simulink model "wind1000_BUS_Original"  (R2024a)
settings: Solver=ode45  StartTime=parSimulacio.ti  StopTime=40  MaxStep=parSimulacio.pas  RelTol=1e-6  AbsTol=1e-6  EnableMultiTasking=on
model workspace (MAT data in the model file):
  tt_vw : Simulink.Parameter (value not decoded)
values from neighbouring files (symbols this model uses):
  L = L'    [parameters_windFarm2.m]
  nu = size(S, 1)    [parameters_windFarm2.m]
undefined symbols (not defined in the file or in its folder): parControl, parConvertidor, parGenerador, parGrid, parMecanica, parSimulacio, S, w0, windspeed
(part 6 of 218)

== system wind1000_BUS_Original/Subsystem_200/Subsystem/String16/WT1 ==
  V_in : Inport
  I_in : Inport  Port=2
  Add1 : Sum  Inputs=+-
  C_cable : Subsystem
  C_filter : Subsystem
  Cable line : Subsystem
  Gain : Gain  Gain=1/parGrid.WTstring.Rparallel_cable
  Transformer-complex : Subsystem
  WFG : Subsystem
  V_out : Outport
  I_out : Outport  Port=2
  wires:
  I_in/1 -> C_cable/2(I_in)
  Gain/1 -> Add1/2
  Add1/1 -> C_cable/1(It2)
  Transformer-complex/2(It2) -> Add1/1
  V_in/1 -> Cable line/1(V)
  Transformer-complex/1(It1) -> C_filter/2(It1)
  Cable line/1(I_cable) -> C_cable/3(I_cable), I_out/1
  C_filter/1(Vc_filter_qd) -> WFG/1(vcqd), Transformer-complex/1(Vc_filter_qd)
  WFG/1(ilqd) -> C_filter/1(IWF)
  C_cable/1(Vc_cable) -> Cable line/2(Vc_cable), V_out/1, Transformer-complex/2(Vc_cable_qd), Gain/1

== system wind1000_BUS_Original/Subsystem_200/Subsystem/String16/WT10 ==
  V_in : Inport
  I_in : Inport  Port=2
  Add1 : Sum  Inputs=+-
  C_cable : Subsystem
  C_filter : Subsystem
  Cable line : Subsystem
  Gain : Gain  Gain=1/parGrid.WTstring.Rparallel_cable
  Transformer-complex : Subsystem
  WFG : Subsystem
  V_out : Outport
  I_out : Outport  Port=2
  wires:
  C_cable/1(Vc_cable) -> Gain/1, Transformer-complex/2(Vc_cable_qd), V_out/1, Cable line/2(Vc_cable)
  WFG/1(ilqd) -> C_filter/1(IWF)
  C_filter/1(Vc_filter_qd) -> Transformer-complex/1(Vc_filter_qd), WFG/1(vcqd)
  Cable line/1(I_cable) -> I_out/1, C_cable/3(I_cable)
  Transformer-complex/1(It1) -> C_filter/2(It1)
  V_in/1 -> Cable line/1(V)
  Transformer-complex/2(It2) -> Add1/1
  Add1/1 -> C_cable/1(It2)
  Gain/1 -> Add1/2
  I_in/1 -> C_cable/2(I_in)

== system wind1000_BUS_Original/Subsystem_200/Subsystem/String16/WT2 ==
  V_in : Inport
  I_in : Inport  Port=2
  Add1 : Sum  Inputs=+-
  C_cable : Subsystem
  C_filter : Subsystem
  Cable line : Subsystem
  Gain : Gain  Gain=1/parGrid.WTstring.Rparallel_cable
  Transformer-complex : Subsystem
  WFG : Subsystem
  V_out : Outport
  I_out : Outport  Port=2
  wires:
  C_cable/1(Vc_cable) -> Gain/1, Transformer-complex/2(Vc_cable_qd), V_out/1, Cable line/2(Vc_cable)
  WFG/1(ilqd) -> C_filter/1(IWF)
  C_filter/1(Vc_filter_qd) -> Transformer-complex/1(Vc_filter_qd), WFG/1(vcqd)
  Cable line/1(I_cable) -> I_out/1, C_cable/3(I_cable)
  Transformer-complex/1(It1) -> C_filter/2(It1)
  V_in/1 -> Cable line/1(V)
  Transformer-complex/2(It2) -> Add1/1
  Add1/1 -> C_cable/1(It2)
  Gain/1 -> Add1/2
  I_in/1 -> C_cable/2(I_in)

== system wind1000_BUS_Original/Subsystem_200/Subsystem/String16/WT3 ==
  V_in : Inport
  I_in : Inport  Port=2
  Add1 : Sum  Inputs=+-
  C_cable : Subsystem
  C_filter : Subsystem
  Cable line : Subsystem
  Gain : Gain  Gain=1/parGrid.WTstring.Rparallel_cable
  Transformer-complex : Subsystem
  WFG : Subsystem
  V_out : Outport
  I_out : Outport  Port=2
  wires:
  I_in/1 -> C_cable/2(I_in)
  Gain/1 -> Add1/2
  Add1/1 -> C_cable/1(It2)
  Transformer-complex/2(It2) -> Add1/1
  V_in/1 -> Cable line/1(V)
  Transformer-complex/1(It1) -> C_filter/2(It1)
  Cable line/1(I_cable) -> C_cable/3(I_cable), I_out/1
  C_filter/1(Vc_filter_qd) -> Transformer-complex/1(Vc_filter_qd), WFG/1(vcqd)
  WFG/1(ilqd) -> C_filter/1(IWF)
  C_cable/1(Vc_cable) -> Cable line/2(Vc_cable), V_out/1, Transformer-complex/2(Vc_cable_qd), Gain/1

== system wind1000_BUS_Original/Subsystem_200/Subsystem/String16/WT4 ==
  V_in : Inport
  I_in : Inport  Port=2
  Add1 : Sum  Inputs=+-
  C_cable : Subsystem
  C_filter : Subsystem
  Cable line : Subsystem
  Gain : Gain  Gain=1/parGrid.WTstring.Rparallel_cable
  Transformer-complex : Subsystem
  WFG : Subsystem
  V_out : Outport
  I_out : Outport  Port=2
  wires:
  C_cable/1(Vc_cable) -> Gain/1, Transformer-complex/2(Vc_cable_qd), V_out/1, Cable line/2(Vc_cable)
  WFG/1(ilqd) -> C_filter/1(IWF)
  C_filter/1(Vc_filter_qd) -> Transformer-complex/1(Vc_filter_qd), WFG/1(vcqd)
  Cable line/1(I_cable) -> I_out/1, C_cable/3(I_cable)
  Transformer-complex/1(It1) -> C_filter/2(It1)
  V_in/1 -> Cable line/1(V)
  Transformer-complex/2(It2) -> Add1/1
  Add1/1 -> C_cable/1(It2)
  Gain/1 -> Add1/2
  I_in/1 -> C_cable/2(I_in)

== system wind1000_BUS_Original/Subsystem_200/Subsystem/String16/WT5 ==
  V_in : Inport
  I_in : Inport  Port=2
  Add1 : Sum  Inputs=+-
  C_cable : Subsystem
  C_filter : Subsystem
  Cable line : Subsystem
  Gain : Gain  Gain=1/parGrid.WTstring.Rparallel_cable
  Transformer-complex : Subsystem
  WFG : Subsystem
  V_out : Outport
  I_out : Outport  Port=2
  wires:
  I_in/1 -> C_cable/2(I_in)
  Gain/1 -> Add1/2
  Add1/1 -> C_cable/1(It2)
  Transformer-complex/2(It2) -> Add1/1
  V_in/1 -> Cable line/1(V)
  Transformer-complex/1(It1) -> C_filter/2(It1)
  Cable line/1(I_cable) -> C_cable/3(I_cable), I_out/1
  C_filter/1(Vc_filter_qd) -> Transformer-complex/1(Vc_filter_qd), WFG/1(vcqd)
  WFG/1(ilqd) -> C_filter/1(IWF)
  C_cable/1(Vc_cable) -> Cable line/2(Vc_cable), V_out/1, Transformer-complex/2(Vc_cable_qd), Gain/1

== system wind1000_BUS_Original/Subsystem_200/Subsystem/String16/WT6 ==
  V_in : Inport
  I_in : Inport  Port=2
  Add1 : Sum  Inputs=+-
  C_cable : Subsystem
  C_filter : Subsystem
  Cable line : Subsystem
  Gain : Gain  Gain=1/parGrid.WTstring.Rparallel_cable
  Transformer-complex : Subsystem
  WFG : Subsystem
  V_out : Outport
  I_out : Outport  Port=2
  wires:
  C_cable/1(Vc_cable) -> Gain/1, Transformer-complex/2(Vc_cable_qd), V_out/1, Cable line/2(Vc_cable)
  WFG/1(ilqd) -> C_filter/1(IWF)
  C_filter/1(Vc_filter_qd) -> Transformer-complex/1(Vc_filter_qd), WFG/1(vcqd)
  Cable line/1(I_cable) -> I_out/1, C_cable/3(I_cable)
  Transformer-complex/1(It1) -> C_filter/2(It1)
  V_in/1 -> Cable line/1(V)
  Transformer-complex/2(It2) -> Add1/1
  Add1/1 -> C_cable/1(It2)
  Gain/1 -> Add1/2
  I_in/1 -> C_cable/2(I_in)

== system wind1000_BUS_Original/Subsystem_200/Subsystem/String16/WT7 ==
  V_in : Inport
  I_in : Inport  Port=2
  Add1 : Sum  Inputs=+-
  C_cable : Subsystem
  C_filter : Subsystem
  Cable line : Subsystem
  Gain : Gain  Gain=1/parGrid.WTstring.Rparallel_cable
  Transformer-complex : Subsystem
  WFG : Subsystem
  V_out : Outport
  I_out : Outport  Port=2
  wires:
  I_in/1 -> C_cable/2(I_in)
  Gain/1 -> Add1/2
  Add1/1 -> C_cable/1(It2)
  Transformer-complex/2(It2) -> Add1/1
  V_in/1 -> Cable line/1(V)
  Transformer-complex/1(It1) -> C_filter/2(It1)
  Cable line/1(I_cable) -> C_cable/3(I_cable), I_out/1
  C_filter/1(Vc_filter_qd) -> Transformer-complex/1(Vc_filter_qd), WFG/1(vcqd)
  WFG/1(ilqd) -> C_filter/1(IWF)
  C_cable/1(Vc_cable) -> Cable line/2(Vc_cable), V_out/1, Transformer-complex/2(Vc_cable_qd), Gain/1

== system wind1000_BUS_Original/Subsystem_200/Subsystem/String16/WT8 ==
  V_in : Inport
  I_in : Inport  Port=2
  Add1 : Sum  Inputs=+-
  C_cable : Subsystem
  C_filter : Subsystem
  Cable line : Subsystem
  Gain : Gain  Gain=1/parGrid.WTstring.Rparallel_cable
  Transformer-complex : Subsystem
  WFG : Subsystem
  V_out : Outport
  I_out : Outport  Port=2
  wires:
  C_cable/1(Vc_cable) -> Gain/1, Transformer-complex/2(Vc_cable_qd), V_out/1, Cable line/2(Vc_cable)
  WFG/1(ilqd) -> C_filter/1(IWF)
  C_filter/1(Vc_filter_qd) -> Transformer-complex/1(Vc_filter_qd), WFG/1(vcqd)
  Cable line/1(I_cable) -> I_out/1, C_cable/3(I_cable)
  Transformer-complex/1(It1) -> C_filter/2(It1)
  V_in/1 -> Cable line/1(V)
  Transformer-complex/2(It2) -> Add1/1
  Add1/1 -> C_cable/1(It2)
  Gain/1 -> Add1/2
  I_in/1 -> C_cable/2(I_in)

== system wind1000_BUS_Original/Subsystem_200/Subsystem/String16/WT9 ==
  V_in : Inport
  I_in : Inport  Port=2
  Add1 : Sum  Inputs=+-
  C_cable : Subsystem
  C_filter : Subsystem
  Cable line : Subsystem
  Gain : Gain  Gain=1/parGrid.WTstring.Rparallel_cable
  Transformer-complex : Subsystem
  WFG : Subsystem
  V_out : Outport
  I_out : Outport  Port=2
  wires:
  I_in/1 -> C_cable/2(I_in)
  Gain/1 -> Add1/2
  Add1/1 -> C_cable/1(It2)
  Transformer-complex/2(It2) -> Add1/1
  V_in/1 -> Cable line/1(V)
  Transformer-complex/1(It1) -> C_filter/2(It1)
  Cable line/1(I_cable) -> C_cable/3(I_cable), I_out/1
  C_filter/1(Vc_filter_qd) -> Transformer-complex/1(Vc_filter_qd), WFG/1(vcqd)
  WFG/1(ilqd) -> C_filter/1(IWF)
  C_cable/1(Vc_cable) -> Cable line/2(Vc_cable), V_out/1, Transformer-complex/2(Vc_cable_qd), Gain/1

== system wind1000_BUS_Original/Subsystem_200/Subsystem/String17/WT1 ==
  V_in : Inport
  I_in : Inport  Port=2
  Add1 : Sum  Inputs=+-
  C_cable : Subsystem
  C_filter : Subsystem
  Cable line : Subsystem
  Gain : Gain  Gain=1/parGrid.WTstring.Rparallel_cable
  Transformer-complex : Subsystem
  WFG : Subsystem
  V_out : Outport
  I_out : Outport  Port=2
  wires:
  I_in/1 -> C_cable/2(I_in)
  Gain/1 -> Add1/2
  Add1/1 -> C_cable/1(It2)
  Transformer-complex/2(It2) -> Add1/1
  V_in/1 -> Cable line/1(V)
  Transformer-complex/1(It1) -> C_filter/2(It1)
  Cable line/1(I_cable) -> C_cable/3(I_cable), I_out/1
  C_filter/1(Vc_filter_qd) -> WFG/1(vcqd), Transformer-complex/1(Vc_filter_qd)
  WFG/1(ilqd) -> C_filter/1(IWF)
  C_cable/1(Vc_cable) -> Cable line/2(Vc_cable), V_out/1, Transformer-complex/2(Vc_cable_qd), Gain/1

== system wind1000_BUS_Original/Subsystem_200/Subsystem/String17/WT10 ==
  V_in : Inport
  I_in : Inport  Port=2
  Add1 : Sum  Inputs=+-
  C_cable : Subsystem
  C_filter : Subsystem
  Cable line : Subsystem
  Gain : Gain  Gain=1/parGrid.WTstring.Rparallel_cable
  Transformer-complex : Subsystem
  WFG : Subsystem
  V_out : Outport
  I_out : Outport  Port=2
  wires:
  C_cable/1(Vc_cable) -> Gain/1, Transformer-complex/2(Vc_cable_qd), V_out/1, Cable line/2(Vc_cable)
  WFG/1(ilqd) -> C_filter/1(IWF)
  C_filter/1(Vc_filter_qd) -> Transformer-complex/1(Vc_filter_qd), WFG/1(vcqd)
  Cable line/1(I_cable) -> I_out/1, C_cable/3(I_cable)
  Transformer-complex/1(It1) -> C_filter/2(It1)
  V_in/1 -> Cable line/1(V)
  Transformer-complex/2(It2) -> Add1/1
  Add1/1 -> C_cable/1(It2)
  Gain/1 -> Add1/2
  I_in/1 -> C_cable/2(I_in)

== system wind1000_BUS_Original/Subsystem_200/Subsystem/String17/WT2 ==
  V_in : Inport
  I_in : Inport  Port=2
  Add1 : Sum  Inputs=+-
  C_cable : Subsystem
  C_filter : Subsystem
  Cable line : Subsystem
  Gain : Gain  Gain=1/parGrid.WTstring.Rparallel_cable
  Transformer-complex : Subsystem
  WFG : Subsystem
  V_out : Outport
  I_out : Outport  Port=2
  wires:
  C_cable/1(Vc_cable) -> Gain/1, Transformer-complex/2(Vc_cable_qd), V_out/1, Cable line/2(Vc_cable)
  WFG/1(ilqd) -> C_filter/1(IWF)
  C_filter/1(Vc_filter_qd) -> Transformer-complex/1(Vc_filter_qd), WFG/1(vcqd)
  Cable line/1(I_cable) -> I_out/1, C_cable/3(I_cable)
  Transformer-complex/1(It1) -> C_filter/2(It1)
  V_in/1 -> Cable line/1(V)
  Transformer-complex/2(It2) -> Add1/1
  Add1/1 -> C_cable/1(It2)
  Gain/1 -> Add1/2
  I_in/1 -> C_cable/2(I_in)

== system wind1000_BUS_Original/Subsystem_200/Subsystem/String17/WT3 ==
  V_in : Inport
  I_in : Inport  Port=2
  Add1 : Sum  Inputs=+-
  C_cable : Subsystem
  C_filter : Subsystem
  Cable line : Subsystem
  Gain : Gain  Gain=1/parGrid.WTstring.Rparallel_cable
  Transformer-complex : Subsystem
  WFG : Subsystem
  V_out : Outport
  I_out : Outport  Port=2
  wires:
  I_in/1 -> C_cable/2(I_in)
  Gain/1 -> Add1/2
  Add1/1 -> C_cable/1(It2)
  Transformer-complex/2(It2) -> Add1/1
  V_in/1 -> Cable line/1(V)
  Transformer-complex/1(It1) -> C_filter/2(It1)
  Cable line/1(I_cable) -> C_cable/3(I_cable), I_out/1
  C_filter/1(Vc_filter_qd) -> Transformer-complex/1(Vc_filter_qd), WFG/1(vcqd)
  WFG/1(ilqd) -> C_filter/1(IWF)
  C_cable/1(Vc_cable) -> Cable line/2(Vc_cable), V_out/1, Transformer-complex/2(Vc_cable_qd), Gain/1

== system wind1000_BUS_Original/Subsystem_200/Subsystem/String17/WT4 ==
  V_in : Inport
  I_in : Inport  Port=2
  Add1 : Sum  Inputs=+-
  C_cable : Subsystem
  C_filter : Subsystem
  Cable line : Subsystem
  Gain : Gain  Gain=1/parGrid.WTstring.Rparallel_cable
  Transformer-complex : Subsystem
  WFG : Subsystem
  V_out : Outport
  I_out : Outport  Port=2
  wires:
  C_cable/1(Vc_cable) -> Gain/1, Transformer-complex/2(Vc_cable_qd), V_out/1, Cable line/2(Vc_cable)
  WFG/1(ilqd) -> C_filter/1(IWF)
  C_filter/1(Vc_filter_qd) -> Transformer-complex/1(Vc_filter_qd), WFG/1(vcqd)
  Cable line/1(I_cable) -> I_out/1, C_cable/3(I_cable)
  Transformer-complex/1(It1) -> C_filter/2(It1)
  V_in/1 -> Cable line/1(V)
  Transformer-complex/2(It2) -> Add1/1
  Add1/1 -> C_cable/1(It2)
  Gain/1 -> Add1/2
  I_in/1 -> C_cable/2(I_in)

== system wind1000_BUS_Original/Subsystem_200/Subsystem/String17/WT5 ==
  V_in : Inport
  I_in : Inport  Port=2
  Add1 : Sum  Inputs=+-
  C_cable : Subsystem
  C_filter : Subsystem
  Cable line : Subsystem
  Gain : Gain  Gain=1/parGrid.WTstring.Rparallel_cable
  Transformer-complex : Subsystem
  WFG : Subsystem
  V_out : Outport
  I_out : Outport  Port=2
  wires:
  I_in/1 -> C_cable/2(I_in)
  Gain/1 -> Add1/2
  Add1/1 -> C_cable/1(It2)
  Transformer-complex/2(It2) -> Add1/1
  V_in/1 -> Cable line/1(V)
  Transformer-complex/1(It1) -> C_filter/2(It1)
  Cable line/1(I_cable) -> C_cable/3(I_cable), I_out/1
  C_filter/1(Vc_filter_qd) -> Transformer-complex/1(Vc_filter_qd), WFG/1(vcqd)
  WFG/1(ilqd) -> C_filter/1(IWF)
  C_cable/1(Vc_cable) -> Cable line/2(Vc_cable), V_out/1, Transformer-complex/2(Vc_cable_qd), Gain/1

== system wind1000_BUS_Original/Subsystem_200/Subsystem/String17/WT6 ==
  V_in : Inport
  I_in : Inport  Port=2
  Add1 : Sum  Inputs=+-
  C_cable : Subsystem
  C_filter : Subsystem
  Cable line : Subsystem
  Gain : Gain  Gain=1/parGrid.WTstring.Rparallel_cable
  Transformer-complex : Subsystem
  WFG : Subsystem
  V_out : Outport
  I_out : Outport  Port=2
  wires:
  C_cable/1(Vc_cable) -> Gain/1, Transformer-complex/2(Vc_cable_qd), V_out/1, Cable line/2(Vc_cable)
  WFG/1(ilqd) -> C_filter/1(IWF)
  C_filter/1(Vc_filter_qd) -> Transformer-complex/1(Vc_filter_qd), WFG/1(vcqd)
  Cable line/1(I_cable) -> I_out/1, C_cable/3(I_cable)
  Transformer-complex/1(It1) -> C_filter/2(It1)
  V_in/1 -> Cable line/1(V)
  Transformer-complex/2(It2) -> Add1/1
  Add1/1 -> C_cable/1(It2)
  Gain/1 -> Add1/2
  I_in/1 -> C_cable/2(I_in)

== system wind1000_BUS_Original/Subsystem_200/Subsystem/String17/WT7 ==
  V_in : Inport
  I_in : Inport  Port=2
  Add1 : Sum  Inputs=+-
  C_cable : Subsystem
  C_filter : Subsystem
  Cable line : Subsystem
  Gain : Gain  Gain=1/parGrid.WTstring.Rparallel_cable
  Transformer-complex : Subsystem
  WFG : Subsystem
  V_out : Outport
  I_out : Outport  Port=2
  wires:
  I_in/1 -> C_cable/2(I_in)
  Gain/1 -> Add1/2
  Add1/1 -> C_cable/1(It2)
  Transformer-complex/2(It2) -> Add1/1
  V_in/1 -> Cable line/1(V)
  Transformer-complex/1(It1) -> C_filter/2(It1)
  Cable line/1(I_cable) -> C_cable/3(I_cable), I_out/1
  C_filter/1(Vc_filter_qd) -> Transformer-complex/1(Vc_filter_qd), WFG/1(vcqd)
  WFG/1(ilqd) -> C_filter/1(IWF)
  C_cable/1(Vc_cable) -> Cable line/2(Vc_cable), V_out/1, Transformer-complex/2(Vc_cable_qd), Gain/1

== system wind1000_BUS_Original/Subsystem_200/Subsystem/String17/WT8 ==
  V_in : Inport
  I_in : Inport  Port=2
  Add1 : Sum  Inputs=+-
  C_cable : Subsystem
  C_filter : Subsystem
  Cable line : Subsystem
  Gain : Gain  Gain=1/parGrid.WTstring.Rparallel_cable
  Transformer-complex : Subsystem
  WFG : Subsystem
  V_out : Outport
  I_out : Outport  Port=2
  wires:
  C_cable/1(Vc_cable) -> Gain/1, Transformer-complex/2(Vc_cable_qd), V_out/1, Cable line/2(Vc_cable)
  WFG/1(ilqd) -> C_filter/1(IWF)
  C_filter/1(Vc_filter_qd) -> Transformer-complex/1(Vc_filter_qd), WFG/1(vcqd)
  Cable line/1(I_cable) -> I_out/1, C_cable/3(I_cable)
  Transformer-complex/1(It1) -> C_filter/2(It1)
  V_in/1 -> Cable line/1(V)
  Transformer-complex/2(It2) -> Add1/1
  Add1/1 -> C_cable/1(It2)
  Gain/1 -> Add1/2
  I_in/1 -> C_cable/2(I_in)

== system wind1000_BUS_Original/Subsystem_200/Subsystem/String17/WT9 ==
  V_in : Inport
  I_in : Inport  Port=2
  Add1 : Sum  Inputs=+-
  C_cable : Subsystem
  C_filter : Subsystem
  Cable line : Subsystem
  Gain : Gain  Gain=1/parGrid.WTstring.Rparallel_cable
  Transformer-complex : Subsystem
  WFG : Subsystem
  V_out : Outport
  I_out : Outport  Port=2
  wires:
  I_in/1 -> C_cable/2(I_in)
  Gain/1 -> Add1/2
  Add1/1 -> C_cable/1(It2)
  Transformer-complex/2(It2) -> Add1/1
  V_in/1 -> Cable line/1(V)
  Transformer-complex/1(It1) -> C_filter/2(It1)
  Cable line/1(I_cable) -> C_cable/3(I_cable), I_out/1
  C_filter/1(Vc_filter_qd) -> Transformer-complex/1(Vc_filter_qd), WFG/1(vcqd)
  WFG/1(ilqd) -> C_filter/1(IWF)
  C_cable/1(Vc_cable) -> Cable line/2(Vc_cable), V_out/1, Transformer-complex/2(Vc_cable_qd), Gain/1

== system wind1000_BUS_Original/Subsystem_200/Subsystem/String18/WT1 ==
  V_in : Inport
  I_in : Inport  Port=2
  Add1 : Sum  Inputs=+-
  C_cable : Subsystem
  C_filter : Subsystem
  Cable line : Subsystem
  Gain : Gain  Gain=1/parGrid.WTstring.Rparallel_cable
  Transformer-complex : Subsystem
  WFG : Subsystem
  V_out : Outport
  I_out : Outport  Port=2
  wires:
  I_in/1 -> C_cable/2(I_in)
  Gain/1 -> Add1/2
  Add1/1 -> C_cable/1(It2)
  Transformer-complex/2(It2) -> Add1/1
  V_in/1 -> Cable line/1(V)
  Transformer-complex/1(It1) -> C_filter/2(It1)
  Cable line/1(I_cable) -> C_cable/3(I_cable), I_out/1
  C_filter/1(Vc_filter_qd) -> WFG/1(vcqd), Transformer-complex/1(Vc_filter_qd)
  WFG/1(ilqd) -> C_filter/1(IWF)
  C_cable/1(Vc_cable) -> Cable line/2(Vc_cable), V_out/1, Transformer-complex/2(Vc_cable_qd), Gain/1

== system wind1000_BUS_Original/Subsystem_200/Subsystem/String18/WT10 ==
  V_in : Inport
  I_in : Inport  Port=2
  Add1 : Sum  Inputs=+-
  C_cable : Subsystem
  C_filter : Subsystem
  Cable line : Subsystem
  Gain : Gain  Gain=1/parGrid.WTstring.Rparallel_cable
  Transformer-complex : Subsystem
  WFG : Subsystem
  V_out : Outport
  I_out : Outport  Port=2
  wires:
  C_cable/1(Vc_cable) -> Gain/1, Transformer-complex/2(Vc_cable_qd), V_out/1, Cable line/2(Vc_cable)
  WFG/1(ilqd) -> C_filter/1(IWF)
  C_filter/1(Vc_filter_qd) -> Transformer-complex/1(Vc_filter_qd), WFG/1(vcqd)
  Cable line/1(I_cable) -> I_out/1, C_cable/3(I_cable)
  Transformer-complex/1(It1) -> C_filter/2(It1)
  V_in/1 -> Cable line/1(V)
  Transformer-complex/2(It2) -> Add1/1
  Add1/1 -> C_cable/1(It2)
  Gain/1 -> Add1/2
  I_in/1 -> C_cable/2(I_in)

== system wind1000_BUS_Original/Subsystem_200/Subsystem/String18/WT2 ==
  V_in : Inport
  I_in : Inport  Port=2
  Add1 : Sum  Inputs=+-
  C_cable : Subsystem
  C_filter : Subsystem
  Cable line : Subsystem
  Gain : Gain  Gain=1/parGrid.WTstring.Rparallel_cable
  Transformer-complex : Subsystem
  WFG : Subsystem
  V_out : Outport
  I_out : Outport  Port=2
  wires:
  C_cable/1(Vc_cable) -> Gain/1, Transformer-complex/2(Vc_cable_qd), V_out/1, Cable line/2(Vc_cable)
  WFG/1(ilqd) -> C_filter/1(IWF)
  C_filter/1(Vc_filter_qd) -> Transformer-complex/1(Vc_filter_qd), WFG/1(vcqd)
  Cable line/1(I_cable) -> I_out/1, C_cable/3(I_cable)
  Transformer-complex/1(It1) -> C_filter/2(It1)
  V_in/1 -> Cable line/1(V)
  Transformer-complex/2(It2) -> Add1/1
  Add1/1 -> C_cable/1(It2)
  Gain/1 -> Add1/2
  I_in/1 -> C_cable/2(I_in)

== system wind1000_BUS_Original/Subsystem_200/Subsystem/String18/WT3 ==
  V_in : Inport
  I_in : Inport  Port=2
  Add1 : Sum  Inputs=+-
  C_cable : Subsystem
  C_filter : Subsystem
  Cable line : Subsystem
  Gain : Gain  Gain=1/parGrid.WTstring.Rparallel_cable
  Transformer-complex : Subsystem
  WFG : Subsystem
  V_out : Outport
  I_out : Outport  Port=2
  wires:
  I_in/1 -> C_cable/2(I_in)
  Gain/1 -> Add1/2
  Add1/1 -> C_cable/1(It2)
  Transformer-complex/2(It2) -> Add1/1
  V_in/1 -> Cable line/1(V)
  Transformer-complex/1(It1) -> C_filter/2(It1)
  Cable line/1(I_cable) -> C_cable/3(I_cable), I_out/1
  C_filter/1(Vc_filter_qd) -> Transformer-complex/1(Vc_filter_qd), WFG/1(vcqd)
  WFG/1(ilqd) -> C_filter/1(IWF)
  C_cable/1(Vc_cable) -> Cable line/2(Vc_cable), V_out/1, Transformer-complex/2(Vc_cable_qd), Gain/1

== system wind1000_BUS_Original/Subsystem_200/Subsystem/String18/WT4 ==
  V_in : Inport
  I_in : Inport  Port=2
  Add1 : Sum  Inputs=+-
  C_cable : Subsystem
  C_filter : Subsystem
  Cable line : Subsystem
  Gain : Gain  Gain=1/parGrid.WTstring.Rparallel_cable
  Transformer-complex : Subsystem
  WFG : Subsystem
  V_out : Outport
  I_out : Outport  Port=2
  wires:
  C_cable/1(Vc_cable) -> Gain/1, Transformer-complex/2(Vc_cable_qd), V_out/1, Cable line/2(Vc_cable)
  WFG/1(ilqd) -> C_filter/1(IWF)
  C_filter/1(Vc_filter_qd) -> Transformer-complex/1(Vc_filter_qd), WFG/1(vcqd)
  Cable line/1(I_cable) -> I_out/1, C_cable/3(I_cable)
  Transformer-complex/1(It1) -> C_filter/2(It1)
  V_in/1 -> Cable line/1(V)
  Transformer-complex/2(It2) -> Add1/1
  Add1/1 -> C_cable/1(It2)
  Gain/1 -> Add1/2
  I_in/1 -> C_cable/2(I_in)

== system wind1000_BUS_Original/Subsystem_200/Subsystem/String18/WT5 ==
  V_in : Inport
  I_in : Inport  Port=2
  Add1 : Sum  Inputs=+-
  C_cable : Subsystem
  C_filter : Subsystem
  Cable line : Subsystem
  Gain : Gain  Gain=1/parGrid.WTstring.Rparallel_cable
  Transformer-complex : Subsystem
  WFG : Subsystem
  V_out : Outport
  I_out : Outport  Port=2
  wires:
  I_in/1 -> C_cable/2(I_in)
  Gain/1 -> Add1/2
  Add1/1 -> C_cable/1(It2)
  Transformer-complex/2(It2) -> Add1/1
  V_in/1 -> Cable line/1(V)
  Transformer-complex/1(It1) -> C_filter/2(It1)
  Cable line/1(I_cable) -> C_cable/3(I_cable), I_out/1
  C_filter/1(Vc_filter_qd) -> Transformer-complex/1(Vc_filter_qd), WFG/1(vcqd)
  WFG/1(ilqd) -> C_filter/1(IWF)
  C_cable/1(Vc_cable) -> Cable line/2(Vc_cable), V_out/1, Transformer-complex/2(Vc_cable_qd), Gain/1

== system wind1000_BUS_Original/Subsystem_200/Subsystem/String18/WT6 ==
  V_in : Inport
  I_in : Inport  Port=2
  Add1 : Sum  Inputs=+-
  C_cable : Subsystem
  C_filter : Subsystem
  Cable line : Subsystem
  Gain : Gain  Gain=1/parGrid.WTstring.Rparallel_cable
  Transformer-complex : Subsystem
  WFG : Subsystem
  V_out : Outport
  I_out : Outport  Port=2
  wires:
  C_cable/1(Vc_cable) -> Gain/1, Transformer-complex/2(Vc_cable_qd), V_out/1, Cable line/2(Vc_cable)
  WFG/1(ilqd) -> C_filter/1(IWF)
  C_filter/1(Vc_filter_qd) -> Transformer-complex/1(Vc_filter_qd), WFG/1(vcqd)
  Cable line/1(I_cable) -> I_out/1, C_cable/3(I_cable)
  Transformer-complex/1(It1) -> C_filter/2(It1)
  V_in/1 -> Cable line/1(V)
  Transformer-complex/2(It2) -> Add1/1
  Add1/1 -> C_cable/1(It2)
  Gain/1 -> Add1/2
  I_in/1 -> C_cable/2(I_in)

== system wind1000_BUS_Original/Subsystem_200/Subsystem/String18/WT7 ==
  V_in : Inport
  I_in : Inport  Port=2
  Add1 : Sum  Inputs=+-
  C_cable : Subsystem
  C_filter : Subsystem
  Cable line : Subsystem
  Gain : Gain  Gain=1/parGrid.WTstring.Rparallel_cable
  Transformer-complex : Subsystem
  WFG : Subsystem
  V_out : Outport
  I_out : Outport  Port=2
  wires:
  I_in/1 -> C_cable/2(I_in)
  Gain/1 -> Add1/2
  Add1/1 -> C_cable/1(It2)
  Transformer-complex/2(It2) -> Add1/1
  V_in/1 -> Cable line/1(V)
  Transformer-complex/1(It1) -> C_filter/2(It1)
  Cable line/1(I_cable) -> C_cable/3(I_cable), I_out/1
  C_filter/1(Vc_filter_qd) -> Transformer-complex/1(Vc_filter_qd), WFG/1(vcqd)
  WFG/1(ilqd) -> C_filter/1(IWF)
  C_cable/1(Vc_cable) -> Cable line/2(Vc_cable), V_out/1, Transformer-complex/2(Vc_cable_qd), Gain/1

== system wind1000_BUS_Original/Subsystem_200/Subsystem/String18/WT8 ==
  V_in : Inport
  I_in : Inport  Port=2
  Add1 : Sum  Inputs=+-
  C_cable : Subsystem
  C_filter : Subsystem
  Cable line : Subsystem
  Gain : Gain  Gain=1/parGrid.WTstring.Rparallel_cable
  Transformer-complex : Subsystem
  WFG : Subsystem
  V_out : Outport
  I_out : Outport  Port=2
  wires:
  C_cable/1(Vc_cable) -> Gain/1, Transformer-complex/2(Vc_cable_qd), V_out/1, Cable line/2(Vc_cable)
  WFG/1(ilqd) -> C_filter/1(IWF)
  C_filter/1(Vc_filter_qd) -> Transformer-complex/1(Vc_filter_qd), WFG/1(vcqd)
  Cable line/1(I_cable) -> I_out/1, C_cable/3(I_cable)
  Transformer-complex/1(It1) -> C_filter/2(It1)
  V_in/1 -> Cable line/1(V)
  Transformer-complex/2(It2) -> Add1/1
  Add1/1 -> C_cable/1(It2)
  Gain/1 -> Add1/2
  I_in/1 -> C_cable/2(I_in)

== system wind1000_BUS_Original/Subsystem_200/Subsystem/String18/WT9 ==
  V_in : Inport
  I_in : Inport  Port=2
  Add1 : Sum  Inputs=+-
  C_cable : Subsystem
  C_filter : Subsystem
  Cable line : Subsystem
  Gain : Gain  Gain=1/parGrid.WTstring.Rparallel_cable
  Transformer-complex : Subsystem
  WFG : Subsystem
  V_out : Outport
  I_out : Outport  Port=2
  wires:
  I_in/1 -> C_cable/2(I_in)
  Gain/1 -> Add1/2
  Add1/1 -> C_cable/1(It2)
  Transformer-complex/2(It2) -> Add1/1
  V_in/1 -> Cable line/1(V)
  Transformer-complex/1(It1) -> C_filter/2(It1)
  Cable line/1(I_cable) -> C_cable/3(I_cable), I_out/1
  C_filter/1(Vc_filter_qd) -> Transformer-complex/1(Vc_filter_qd), WFG/1(vcqd)
  WFG/1(ilqd) -> C_filter/1(IWF)
  C_cable/1(Vc_cable) -> Cable line/2(Vc_cable), V_out/1, Transformer-complex/2(Vc_cable_qd), Gain/1

== system wind1000_BUS_Original/Subsystem_200/Subsystem/String19/WT1 ==
  V_in : Inport
  I_in : Inport  Port=2
  Add1 : Sum  Inputs=+-
  C_cable : Subsystem
  C_filter : Subsystem
  Cable line : Subsystem
  Gain : Gain  Gain=1/parGrid.WTstring.Rparallel_cable
  Transformer-complex : Subsystem
  WFG : Subsystem
  V_out : Outport
  I_out : Outport  Port=2
  wires:
  I_in/1 -> C_cable/2(I_in)
  Gain/1 -> Add1/2
  Add1/1 -> C_cable/1(It2)
  Transformer-complex/2(It2) -> Add1/1
  V_in/1 -> Cable line/1(V)
  Transformer-complex/1(It1) -> C_filter/2(It1)
  Cable line/1(I_cable) -> C_cable/3(I_cable), I_out/1
  C_filter/1(Vc_filter_qd) -> WFG/1(vcqd), Transformer-complex/1(Vc_filter_qd)
  WFG/1(ilqd) -> C_filter/1(IWF)
  C_cable/1(Vc_cable) -> Cable line/2(Vc_cable), V_out/1, Transformer-complex/2(Vc_cable_qd), Gain/1

== system wind1000_BUS_Original/Subsystem_200/Subsystem/String19/WT10 ==
  V_in : Inport
  I_in : Inport  Port=2
  Add1 : Sum  Inputs=+-
  C_cable : Subsystem
  C_filter : Subsystem
  Cable line : Subsystem
  Gain : Gain  Gain=1/parGrid.WTstring.Rparallel_cable
  Transformer-complex : Subsystem
  WFG : Subsystem
  V_out : Outport
  I_out : Outport  Port=2
  wires:
  C_cable/1(Vc_cable) -> Gain/1, Transformer-complex/2(Vc_cable_qd), V_out/1, Cable line/2(Vc_cable)
  WFG/1(ilqd) -> C_filter/1(IWF)
  C_filter/1(Vc_filter_qd) -> Transformer-complex/1(Vc_filter_qd), WFG/1(vcqd)
  Cable line/1(I_cable) -> I_out/1, C_cable/3(I_cable)
  Transformer-complex/1(It1) -> C_filter/2(It1)
  V_in/1 -> Cable line/1(V)
  Transformer-complex/2(It2) -> Add1/1
  Add1/1 -> C_cable/1(It2)
  Gain/1 -> Add1/2
  I_in/1 -> C_cable/2(I_in)

== system wind1000_BUS_Original/Subsystem_200/Subsystem/String19/WT2 ==
  V_in : Inport
  I_in : Inport  Port=2
  Add1 : Sum  Inputs=+-
  C_cable : Subsystem
  C_filter : Subsystem
  Cable line : Subsystem
  Gain : Gain  Gain=1/parGrid.WTstring.Rparallel_cable
  Transformer-complex : Subsystem
  WFG : Subsystem
  V_out : Outport
  I_out : Outport  Port=2
  wires:
  C_cable/1(Vc_cable) -> Gain/1, Transformer-complex/2(Vc_cable_qd), V_out/1, Cable line/2(Vc_cable)
  WFG/1(ilqd) -> C_filter/1(IWF)
  C_filter/1(Vc_filter_qd) -> Transformer-complex/1(Vc_filter_qd), WFG/1(vcqd)
  Cable line/1(I_cable) -> I_out/1, C_cable/3(I_cable)
  Transformer-complex/1(It1) -> C_filter/2(It1)
  V_in/1 -> Cable line/1(V)
  Transformer-complex/2(It2) -> Add1/1
  Add1/1 -> C_cable/1(It2)
  Gain/1 -> Add1/2
  I_in/1 -> C_cable/2(I_in)

== system wind1000_BUS_Original/Subsystem_200/Subsystem/String19/WT3 ==
  V_in : Inport
  I_in : Inport  Port=2
  Add1 : Sum  Inputs=+-
  C_cable : Subsystem
  C_filter : Subsystem
  Cable line : Subsystem
  Gain : Gain  Gain=1/parGrid.WTstring.Rparallel_cable
  Transformer-complex : Subsystem
  WFG : Subsystem
  V_out : Outport
  I_out : Outport  Port=2
  wires:
  I_in/1 -> C_cable/2(I_in)
  Gain/1 -> Add1/2
  Add1/1 -> C_cable/1(It2)
  Transformer-complex/2(It2) -> Add1/1
  V_in/1 -> Cable line/1(V)
  Transformer-complex/1(It1) -> C_filter/2(It1)
  Cable line/1(I_cable) -> C_cable/3(I_cable), I_out/1
  C_filter/1(Vc_filter_qd) -> Transformer-complex/1(Vc_filter_qd), WFG/1(vcqd)
  WFG/1(ilqd) -> C_filter/1(IWF)
  C_cable/1(Vc_cable) -> Cable line/2(Vc_cable), V_out/1, Transformer-complex/2(Vc_cable_qd), Gain/1

== system wind1000_BUS_Original/Subsystem_200/Subsystem/String19/WT4 ==
  V_in : Inport
  I_in : Inport  Port=2
  Add1 : Sum  Inputs=+-
  C_cable : Subsystem
  C_filter : Subsystem
  Cable line : Subsystem
  Gain : Gain  Gain=1/parGrid.WTstring.Rparallel_cable
  Transformer-complex : Subsystem
  WFG : Subsystem
  V_out : Outport
  I_out : Outport  Port=2
  wires:
  C_cable/1(Vc_cable) -> Gain/1, Transformer-complex/2(Vc_cable_qd), V_out/1, Cable line/2(Vc_cable)
  WFG/1(ilqd) -> C_filter/1(IWF)
  C_filter/1(Vc_filter_qd) -> Transformer-complex/1(Vc_filter_qd), WFG/1(vcqd)
  Cable line/1(I_cable) -> I_out/1, C_cable/3(I_cable)
  Transformer-complex/1(It1) -> C_filter/2(It1)
  V_in/1 -> Cable line/1(V)
  Transformer-complex/2(It2) -> Add1/1
  Add1/1 -> C_cable/1(It2)
  Gain/1 -> Add1/2
  I_in/1 -> C_cable/2(I_in)

== system wind1000_BUS_Original/Subsystem_200/Subsystem/String19/WT5 ==
  V_in : Inport
  I_in : Inport  Port=2
  Add1 : Sum  Inputs=+-
  C_cable : Subsystem
  C_filter : Subsystem
  Cable line : Subsystem
  Gain : Gain  Gain=1/parGrid.WTstring.Rparallel_cable
  Transformer-complex : Subsystem
  WFG : Subsystem
  V_out : Outport
  I_out : Outport  Port=2
  wires:
  I_in/1 -> C_cable/2(I_in)
  Gain/1 -> Add1/2
  Add1/1 -> C_cable/1(It2)
  Transformer-complex/2(It2) -> Add1/1
  V_in/1 -> Cable line/1(V)
  Transformer-complex/1(It1) -> C_filter/2(It1)
  Cable line/1(I_cable) -> C_cable/3(I_cable), I_out/1
  C_filter/1(Vc_filter_qd) -> Transformer-complex/1(Vc_filter_qd), WFG/1(vcqd)
  WFG/1(ilqd) -> C_filter/1(IWF)
  C_cable/1(Vc_cable) -> Cable line/2(Vc_cable), V_out/1, Transformer-complex/2(Vc_cable_qd), Gain/1

== system wind1000_BUS_Original/Subsystem_200/Subsystem/String19/WT6 ==
  V_in : Inport
  I_in : Inport  Port=2
  Add1 : Sum  Inputs=+-
  C_cable : Subsystem
  C_filter : Subsystem
  Cable line : Subsystem
  Gain : Gain  Gain=1/parGrid.WTstring.Rparallel_cable
  Transformer-complex : Subsystem
  WFG : Subsystem
  V_out : Outport
  I_out : Outport  Port=2
  wires:
  C_cable/1(Vc_cable) -> Gain/1, Transformer-complex/2(Vc_cable_qd), V_out/1, Cable line/2(Vc_cable)
  WFG/1(ilqd) -> C_filter/1(IWF)
  C_filter/1(Vc_filter_qd) -> Transformer-complex/1(Vc_filter_qd), WFG/1(vcqd)
  Cable line/1(I_cable) -> I_out/1, C_cable/3(I_cable)
  Transformer-complex/1(It1) -> C_filter/2(It1)
  V_in/1 -> Cable line/1(V)
  Transformer-complex/2(It2) -> Add1/1
  Add1/1 -> C_cable/1(It2)
  Gain/1 -> Add1/2
  I_in/1 -> C_cable/2(I_in)

== system wind1000_BUS_Original/Subsystem_200/Subsystem/String19/WT7 ==
  V_in : Inport
  I_in : Inport  Port=2
  Add1 : Sum  Inputs=+-
  C_cable : Subsystem
  C_filter : Subsystem
  Cable line : Subsystem
  Gain : Gain  Gain=1/parGrid.WTstring.Rparallel_cable
  Transformer-complex : Subsystem
  WFG : Subsystem
  V_out : Outport
  I_out : Outport  Port=2
  wires:
  I_in/1 -> C_cable/2(I_in)
  Gain/1 -> Add1/2
  Add1/1 -> C_cable/1(It2)
  Transformer-complex/2(It2) -> Add1/1
  V_in/1 -> Cable line/1(V)
  Transformer-complex/1(It1) -> C_filter/2(It1)
  Cable line/1(I_cable) -> C_cable/3(I_cable), I_out/1
  C_filter/1(Vc_filter_qd) -> Transformer-complex/1(Vc_filter_qd), WFG/1(vcqd)
  WFG/1(ilqd) -> C_filter/1(IWF)
  C_cable/1(Vc_cable) -> Cable line/2(Vc_cable), V_out/1, Transformer-complex/2(Vc_cable_qd), Gain/1

== system wind1000_BUS_Original/Subsystem_200/Subsystem/String19/WT8 ==
  V_in : Inport
  I_in : Inport  Port=2
  Add1 : Sum  Inputs=+-
  C_cable : Subsystem
  C_filter : Subsystem
  Cable line : Subsystem
  Gain : Gain  Gain=1/parGrid.WTstring.Rparallel_cable
  Transformer-complex : Subsystem
  WFG : Subsystem
  V_out : Outport
  I_out : Outport  Port=2
  wires:
  C_cable/1(Vc_cable) -> Gain/1, Transformer-complex/2(Vc_cable_qd), V_out/1, Cable line/2(Vc_cable)
  WFG/1(ilqd) -> C_filter/1(IWF)
  C_filter/1(Vc_filter_qd) -> Transformer-complex/1(Vc_filter_qd), WFG/1(vcqd)
  Cable line/1(I_cable) -> I_out/1, C_cable/3(I_cable)
  Transformer-complex/1(It1) -> C_filter/2(It1)
  V_in/1 -> Cable line/1(V)
  Transformer-complex/2(It2) -> Add1/1
  Add1/1 -> C_cable/1(It2)
  Gain/1 -> Add1/2
  I_in/1 -> C_cable/2(I_in)

== system wind1000_BUS_Original/Subsystem_200/Subsystem/String19/WT9 ==
  V_in : Inport
  I_in : Inport  Port=2
  Add1 : Sum  Inputs=+-
  C_cable : Subsystem
  C_filter : Subsystem
  Cable line : Subsystem
  Gain : Gain  Gain=1/parGrid.WTstring.Rparallel_cable
  Transformer-complex : Subsystem
  WFG : Subsystem
  V_out : Outport
  I_out : Outport  Port=2
  wires:
  I_in/1 -> C_cable/2(I_in)
  Gain/1 -> Add1/2
  Add1/1 -> C_cable/1(It2)
  Transformer-complex/2(It2) -> Add1/1
  V_in/1 -> Cable line/1(V)
  Transformer-complex/1(It1) -> C_filter/2(It1)
  Cable line/1(I_cable) -> C_cable/3(I_cable), I_out/1
  C_filter/1(Vc_filter_qd) -> Transformer-complex/1(Vc_filter_qd), WFG/1(vcqd)
  WFG/1(ilqd) -> C_filter/1(IWF)
  C_cable/1(Vc_cable) -> Cable line/2(Vc_cable), V_out/1, Transformer-complex/2(Vc_cable_qd), Gain/1

== system wind1000_BUS_Original/Subsystem_200/Subsystem/String2/WT1 ==
  V_in : Inport
  I_in : Inport  Port=2
  Add1 : Sum  Inputs=+-
  C_cable : Subsystem
  C_filter : Subsystem
  Cable line : Subsystem
  Gain : Gain  Gain=1/parGrid.WTstring.Rparallel_cable
  Transformer-complex : Subsystem
  WFG : Subsystem
  V_out : Outport
  I_out : Outport  Port=2
  wires:
  I_in/1 -> C_cable/2(I_in)
  Gain/1 -> Add1/2
  Add1/1 -> C_cable/1(It2)
  Transformer-complex/2(It2) -> Add1/1
  V_in/1 -> Cable line/1(V)
  Transformer-complex/1(It1) -> C_filter/2(It1)
  Cable line/1(I_cable) -> C_cable/3(I_cable), I_out/1
  C_filter/1(Vc_filter_qd) -> WFG/1(vcqd), Transformer-complex/1(Vc_filter_qd)
  WFG/1(ilqd) -> C_filter/1(IWF)
  C_cable/1(Vc_cable) -> Cable line/2(Vc_cable), V_out/1, Transformer-complex/2(Vc_cable_qd), Gain/1

== system wind1000_BUS_Original/Subsystem_200/Subsystem/String2/WT10 ==
  V_in : Inport
  I_in : Inport  Port=2
  Add1 : Sum  Inputs=+-
  C_cable : Subsystem
  C_filter : Subsystem
  Cable line : Subsystem
  Gain : Gain  Gain=1/parGrid.WTstring.Rparallel_cable
  Transformer-complex : Subsystem
  WFG : Subsystem
  V_out : Outport
  I_out : Outport  Port=2
  wires:
  C_cable/1(Vc_cable) -> Gain/1, Transformer-complex/2(Vc_cable_qd), V_out/1, Cable line/2(Vc_cable)
  WFG/1(ilqd) -> C_filter/1(IWF)
  C_filter/1(Vc_filter_qd) -> Transformer-complex/1(Vc_filter_qd), WFG/1(vcqd)
  Cable line/1(I_cable) -> I_out/1, C_cable/3(I_cable)
  Transformer-complex/1(It1) -> C_filter/2(It1)
  V_in/1 -> Cable line/1(V)
  Transformer-complex/2(It2) -> Add1/1
  Add1/1 -> C_cable/1(It2)
  Gain/1 -> Add1/2
  I_in/1 -> C_cable/2(I_in)

== system wind1000_BUS_Original/Subsystem_200/Subsystem/String2/WT2 ==
  V_in : Inport
  I_in : Inport  Port=2
  Add1 : Sum  Inputs=+-
  C_cable : Subsystem
  C_filter : Subsystem
  Cable line : Subsystem
  Gain : Gain  Gain=1/parGrid.WTstring.Rparallel_cable
  Transformer-complex : Subsystem
  WFG : Subsystem
  V_out : Outport
  I_out : Outport  Port=2
  wires:
  C_cable/1(Vc_cable) -> Gain/1, Transformer-complex/2(Vc_cable_qd), V_out/1, Cable line/2(Vc_cable)
  WFG/1(ilqd) -> C_filter/1(IWF)
  C_filter/1(Vc_filter_qd) -> Transformer-complex/1(Vc_filter_qd), WFG/1(vcqd)
  Cable line/1(I_cable) -> I_out/1, C_cable/3(I_cable)
  Transformer-complex/1(It1) -> C_filter/2(It1)
  V_in/1 -> Cable line/1(V)
  Transformer-complex/2(It2) -> Add1/1
  Add1/1 -> C_cable/1(It2)
  Gain/1 -> Add1/2
  I_in/1 -> C_cable/2(I_in)

== system wind1000_BUS_Original/Subsystem_200/Subsystem/String2/WT3 ==
  V_in : Inport
  I_in : Inport  Port=2
  Add1 : Sum  Inputs=+-
  C_cable : Subsystem
  C_filter : Subsystem
  Cable line : Subsystem
  Gain : Gain  Gain=1/parGrid.WTstring.Rparallel_cable
  Transformer-complex : Subsystem
  WFG : Subsystem
  V_out : Outport
  I_out : Outport  Port=2
  wires:
  I_in/1 -> C_cable/2(I_in)
  Gain/1 -> Add1/2
  Add1/1 -> C_cable/1(It2)
  Transformer-complex/2(It2) -> Add1/1
  V_in/1 -> Cable line/1(V)
  Transformer-complex/1(It1) -> C_filter/2(It1)
  Cable line/1(I_cable) -> C_cable/3(I_cable), I_out/1
  C_filter/1(Vc_filter_qd) -> Transformer-complex/1(Vc_filter_qd), WFG/1(vcqd)
  WFG/1(ilqd) -> C_filter/1(IWF)
  C_cable/1(Vc_cable) -> Cable line/2(Vc_cable), V_out/1, Transformer-complex/2(Vc_cable_qd), Gain/1

== system wind1000_BUS_Original/Subsystem_200/Subsystem/String2/WT4 ==
  V_in : Inport
  I_in : Inport  Port=2
  Add1 : Sum  Inputs=+-
  C_cable : Subsystem
  C_filter : Subsystem
  Cable line : Subsystem
  Gain : Gain  Gain=1/parGrid.WTstring.Rparallel_cable
  Transformer-complex : Subsystem
  WFG : Subsystem
  V_out : Outport
  I_out : Outport  Port=2
  wires:
  C_cable/1(Vc_cable) -> Gain/1, Transformer-complex/2(Vc_cable_qd), V_out/1, Cable line/2(Vc_cable)
  WFG/1(ilqd) -> C_filter/1(IWF)
  C_filter/1(Vc_filter_qd) -> Transformer-complex/1(Vc_filter_qd), WFG/1(vcqd)
  Cable line/1(I_cable) -> I_out/1, C_cable/3(I_cable)
  Transformer-complex/1(It1) -> C_filter/2(It1)
  V_in/1 -> Cable line/1(V)
  Transformer-complex/2(It2) -> Add1/1
  Add1/1 -> C_cable/1(It2)
  Gain/1 -> Add1/2
  I_in/1 -> C_cable/2(I_in)

== system wind1000_BUS_Original/Subsystem_200/Subsystem/String2/WT5 ==
  V_in : Inport
  I_in : Inport  Port=2
  Add1 : Sum  Inputs=+-
  C_cable : Subsystem
  C_filter : Subsystem
  Cable line : Subsystem
  Gain : Gain  Gain=1/parGrid.WTstring.Rparallel_cable
  Transformer-complex : Subsystem
  WFG : Subsystem
  V_out : Outport
  I_out : Outport  Port=2
  wires:
  I_in/1 -> C_cable/2(I_in)
  Gain/1 -> Add1/2
  Add1/1 -> C_cable/1(It2)
  Transformer-complex/2(It2) -> Add1/1
  V_in/1 -> Cable line/1(V)
  Transformer-complex/1(It1) -> C_filter/2(It1)
  Cable line/1(I_cable) -> C_cable/3(I_cable), I_out/1
  C_filter/1(Vc_filter_qd) -> Transformer-complex/1(Vc_filter_qd), WFG/1(vcqd)
  WFG/1(ilqd) -> C_filter/1(IWF)
  C_cable/1(Vc_cable) -> Cable line/2(Vc_cable), V_out/1, Transformer-complex/2(Vc_cable_qd), Gain/1

== system wind1000_BUS_Original/Subsystem_200/Subsystem/String2/WT6 ==
  V_in : Inport
  I_in : Inport  Port=2
  Add1 : Sum  Inputs=+-
  C_cable : Subsystem
  C_filter : Subsystem
  Cable line : Subsystem
  Gain : Gain  Gain=1/parGrid.WTstring.Rparallel_cable
  Transformer-complex : Subsystem
  WFG : Subsystem
  V_out : Outport
  I_out : Outport  Port=2
  wires:
  C_cable/1(Vc_cable) -> Gain/1, Transformer-complex/2(Vc_cable_qd), V_out/1, Cable line/2(Vc_cable)
  WFG/1(ilqd) -> C_filter/1(IWF)
  C_filter/1(Vc_filter_qd) -> Transformer-complex/1(Vc_filter_qd), WFG/1(vcqd)
  Cable line/1(I_cable) -> I_out/1, C_cable/3(I_cable)
  Transformer-complex/1(It1) -> C_filter/2(It1)
  V_in/1 -> Cable line/1(V)
  Transformer-complex/2(It2) -> Add1/1
  Add1/1 -> C_cable/1(It2)
  Gain/1 -> Add1/2
  I_in/1 -> C_cable/2(I_in)

== system wind1000_BUS_Original/Subsystem_200/Subsystem/String2/WT7 ==
  V_in : Inport
  I_in : Inport  Port=2
  Add1 : Sum  Inputs=+-
  C_cable : Subsystem
  C_filter : Subsystem
  Cable line : Subsystem
  Gain : Gain  Gain=1/parGrid.WTstring.Rparallel_cable
  Transformer-complex : Subsystem
  WFG : Subsystem
  V_out : Outport
  I_out : Outport  Port=2
  wires:
  I_in/1 -> C_cable/2(I_in)
  Gain/1 -> Add1/2
  Add1/1 -> C_cable/1(It2)
  Transformer-complex/2(It2) -> Add1/1
  V_in/1 -> Cable line/1(V)
  Transformer-complex/1(It1) -> C_filter/2(It1)
  Cable line/1(I_cable) -> C_cable/3(I_cable), I_out/1
  C_filter/1(Vc_filter_qd) -> Transformer-complex/1(Vc_filter_qd), WFG/1(vcqd)
  WFG/1(ilqd) -> C_filter/1(IWF)
  C_cable/1(Vc_cable) -> Cable line/2(Vc_cable), V_out/1, Transformer-complex/2(Vc_cable_qd), Gain/1

== system wind1000_BUS_Original/Subsystem_200/Subsystem/String2/WT8 ==
  V_in : Inport
  I_in : Inport  Port=2
  Add1 : Sum  Inputs=+-
  C_cable : Subsystem
  C_filter : Subsystem
  Cable line : Subsystem
  Gain : Gain  Gain=1/parGrid.WTstring.Rparallel_cable
  Transformer-complex : Subsystem
  WFG : Subsystem
  V_out : Outport
  I_out : Outport  Port=2
  wires:
  C_cable/1(Vc_cable) -> Gain/1, Transformer-complex/2(Vc_cable_qd), V_out/1, Cable line/2(Vc_cable)
  WFG/1(ilqd) -> C_filter/1(IWF)
  C_filter/1(Vc_filter_qd) -> Transformer-complex/1(Vc_filter_qd), WFG/1(vcqd)
  Cable line/1(I_cable) -> I_out/1, C_cable/3(I_cable)
  Transformer-complex/1(It1) -> C_filter/2(It1)
  V_in/1 -> Cable line/1(V)
  Transformer-complex/2(It2) -> Add1/1
  Add1/1 -> C_cable/1(It2)
  Gain/1 -> Add1/2
  I_in/1 -> C_cable/2(I_in)

== system wind1000_BUS_Original/Subsystem_200/Subsystem/String2/WT9 ==
  V_in : Inport
  I_in : Inport  Port=2
  Add1 : Sum  Inputs=+-
  C_cable : Subsystem
  C_filter : Subsystem
  Cable line : Subsystem
  Gain : Gain  Gain=1/parGrid.WTstring.Rparallel_cable
  Transformer-complex : Subsystem
  WFG : Subsystem
  V_out : Outport
  I_out : Outport  Port=2
  wires:
  I_in/1 -> C_cable/2(I_in)
  Gain/1 -> Add1/2
  Add1/1 -> C_cable/1(It2)
  Transformer-complex/2(It2) -> Add1/1
  V_in/1 -> Cable line/1(V)
  Transformer-complex/1(It1) -> C_filter/2(It1)
  Cable line/1(I_cable) -> C_cable/3(I_cable), I_out/1
  C_filter/1(Vc_filter_qd) -> Transformer-complex/1(Vc_filter_qd), WFG/1(vcqd)
  WFG/1(ilqd) -> C_filter/1(IWF)
  C_cable/1(Vc_cable) -> Cable line/2(Vc_cable), V_out/1, Transformer-complex/2(Vc_cable_qd), Gain/1

== system wind1000_BUS_Original/Subsystem_200/Subsystem/String20/WT1 ==
  V_in : Inport
  I_in : Inport  Port=2
  Add1 : Sum  Inputs=+-
  C_cable : Subsystem
  C_filter : Subsystem
  Cable line : Subsystem
  Gain : Gain  Gain=1/parGrid.WTstring.Rparallel_cable
  Transformer-complex : Subsystem
  WFG : Subsystem
  V_out : Outport
  I_out : Outport  Port=2
  wires:
  I_in/1 -> C_cable/2(I_in)
  Gain/1 -> Add1/2
  Add1/1 -> C_cable/1(It2)
  Transformer-complex/2(It2) -> Add1/1
  V_in/1 -> Cable line/1(V)
  Transformer-complex/1(It1) -> C_filter/2(It1)
  Cable line/1(I_cable) -> C_cable/3(I_cable), I_out/1
  C_filter/1(Vc_filter_qd) -> WFG/1(vcqd), Transformer-complex/1(Vc_filter_qd)
  WFG/1(ilqd) -> C_filter/1(IWF)
  C_cable/1(Vc_cable) -> Cable line/2(Vc_cable), V_out/1, Transformer-complex/2(Vc_cable_qd), Gain/1

== system wind1000_BUS_Original/Subsystem_200/Subsystem/String20/WT10 ==
  V_in : Inport
  I_in : Inport  Port=2
  Add1 : Sum  Inputs=+-
  C_cable : Subsystem
  C_filter : Subsystem
  Cable line : Subsystem
  Gain : Gain  Gain=1/parGrid.WTstring.Rparallel_cable
  Transformer-complex : Subsystem
  WFG : Subsystem
  V_out : Outport
  I_out : Outport  Port=2
  wires:
  C_cable/1(Vc_cable) -> Gain/1, Transformer-complex/2(Vc_cable_qd), V_out/1, Cable line/2(Vc_cable)
  WFG/1(ilqd) -> C_filter/1(IWF)
  C_filter/1(Vc_filter_qd) -> Transformer-complex/1(Vc_filter_qd), WFG/1(vcqd)
  Cable line/1(I_cable) -> I_out/1, C_cable/3(I_cable)
  Transformer-complex/1(It1) -> C_filter/2(It1)
  V_in/1 -> Cable line/1(V)
  Transformer-complex/2(It2) -> Add1/1
  Add1/1 -> C_cable/1(It2)
  Gain/1 -> Add1/2
  I_in/1 -> C_cable/2(I_in)

== system wind1000_BUS_Original/Subsystem_200/Subsystem/String20/WT2 ==
  V_in : Inport
  I_in : Inport  Port=2
  Add1 : Sum  Inputs=+-
  C_cable : Subsystem
  C_filter : Subsystem
  Cable line : Subsystem
  Gain : Gain  Gain=1/parGrid.WTstring.Rparallel_cable
  Transformer-complex : Subsystem
  WFG : Subsystem
  V_out : Outport
  I_out : Outport  Port=2
  wires:
  C_cable/1(Vc_cable) -> Gain/1, Transformer-complex/2(Vc_cable_qd), V_out/1, Cable line/2(Vc_cable)
  WFG/1(ilqd) -> C_filter/1(IWF)
  C_filter/1(Vc_filter_qd) -> Transformer-complex/1(Vc_filter_qd), WFG/1(vcqd)
  Cable line/1(I_cable) -> I_out/1, C_cable/3(I_cable)
  Transformer-complex/1(It1) -> C_filter/2(It1)
  V_in/1 -> Cable line/1(V)
  Transformer-complex/2(It2) -> Add1/1
  Add1/1 -> C_cable/1(It2)
  Gain/1 -> Add1/2
  I_in/1 -> C_cable/2(I_in)

== system wind1000_BUS_Original/Subsystem_200/Subsystem/String20/WT3 ==
  V_in : Inport
  I_in : Inport  Port=2
  Add1 : Sum  Inputs=+-
  C_cable : Subsystem
  C_filter : Subsystem
  Cable line : Subsystem
  Gain : Gain  Gain=1/parGrid.WTstring.Rparallel_cable
  Transformer-complex : Subsystem
  WFG : Subsystem
  V_out : Outport
  I_out : Outport  Port=2
  wires:
  I_in/1 -> C_cable/2(I_in)
  Gain/1 -> Add1/2
  Add1/1 -> C_cable/1(It2)
  Transformer-complex/2(It2) -> Add1/1
  V_in/1 -> Cable line/1(V)
  Transformer-complex/1(It1) -> C_filter/2(It1)
  Cable line/1(I_cable) -> C_cable/3(I_cable), I_out/1
  C_filter/1(Vc_filter_qd) -> Transformer-complex/1(Vc_filter_qd), WFG/1(vcqd)
  WFG/1(ilqd) -> C_filter/1(IWF)
  C_cable/1(Vc_cable) -> Cable line/2(Vc_cable), V_out/1, Transformer-complex/2(Vc_cable_qd), Gain/1

== system wind1000_BUS_Original/Subsystem_200/Subsystem/String20/WT4 ==
  V_in : Inport
  I_in : Inport  Port=2
  Add1 : Sum  Inputs=+-
  C_cable : Subsystem
  C_filter : Subsystem
  Cable line : Subsystem
  Gain : Gain  Gain=1/parGrid.WTstring.Rparallel_cable
  Transformer-complex : Subsystem
  WFG : Subsystem
  V_out : Outport
  I_out : Outport  Port=2
  wires:
  C_cable/1(Vc_cable) -> Gain/1, Transformer-complex/2(Vc_cable_qd), V_out/1, Cable line/2(Vc_cable)
  WFG/1(ilqd) -> C_filter/1(IWF)
  C_filter/1(Vc_filter_qd) -> Transformer-complex/1(Vc_filter_qd), WFG/1(vcqd)
  Cable line/1(I_cable) -> I_out/1, C_cable/3(I_cable)
  Transformer-complex/1(It1) -> C_filter/2(It1)
  V_in/1 -> Cable line/1(V)
  Transformer-complex/2(It2) -> Add1/1
  Add1/1 -> C_cable/1(It2)
  Gain/1 -> Add1/2
  I_in/1 -> C_cable/2(I_in)

== system wind1000_BUS_Original/Subsystem_200/Subsystem/String20/WT5 ==
  V_in : Inport
  I_in : Inport  Port=2
  Add1 : Sum  Inputs=+-
  C_cable : Subsystem
  C_filter : Subsystem
  Cable line : Subsystem
  Gain : Gain  Gain=1/parGrid.WTstring.Rparallel_cable
  Transformer-complex : Subsystem
  WFG : Subsystem
  V_out : Outport
  I_out : Outport  Port=2
  wires:
  I_in/1 -> C_cable/2(I_in)
  Gain/1 -> Add1/2
  Add1/1 -> C_cable/1(It2)
  Transformer-complex/2(It2) -> Add1/1
  V_in/1 -> Cable line/1(V)
  Transformer-complex/1(It1) -> C_filter/2(It1)
  Cable line/1(I_cable) -> C_cable/3(I_cable), I_out/1
  C_filter/1(Vc_filter_qd) -> Transformer-complex/1(Vc_filter_qd), WFG/1(vcqd)
  WFG/1(ilqd) -> C_filter/1(IWF)
  C_cable/1(Vc_cable) -> Cable line/2(Vc_cable), V_out/1, Transformer-complex/2(Vc_cable_qd), Gain/1

== system wind1000_BUS_Original/Subsystem_200/Subsystem/String20/WT6 ==
  V_in : Inport
  I_in : Inport  Port=2
  Add1 : Sum  Inputs=+-
  C_cable : Subsystem
  C_filter : Subsystem
  Cable line : Subsystem
  Gain : Gain  Gain=1/parGrid.WTstring.Rparallel_cable
  Transformer-complex : Subsystem
  WFG : Subsystem
  V_out : Outport
  I_out : Outport  Port=2
  wires:
  C_cable/1(Vc_cable) -> Gain/1, Transformer-complex/2(Vc_cable_qd), V_out/1, Cable line/2(Vc_cable)
  WFG/1(ilqd) -> C_filter/1(IWF)
  C_filter/1(Vc_filter_qd) -> Transformer-complex/1(Vc_filter_qd), WFG/1(vcqd)
  Cable line/1(I_cable) -> I_out/1, C_cable/3(I_cable)
  Transformer-complex/1(It1) -> C_filter/2(It1)
  V_in/1 -> Cable line/1(V)
  Transformer-complex/2(It2) -> Add1/1
  Add1/1 -> C_cable/1(It2)
  Gain/1 -> Add1/2
  I_in/1 -> C_cable/2(I_in)

== system wind1000_BUS_Original/Subsystem_200/Subsystem/String20/WT7 ==
  V_in : Inport
  I_in : Inport  Port=2
  Add1 : Sum  Inputs=+-
  C_cable : Subsystem
  C_filter : Subsystem
  Cable line : Subsystem
  Gain : Gain  Gain=1/parGrid.WTstring.Rparallel_cable
  Transformer-complex : Subsystem
  WFG : Subsystem
  V_out : Outport
  I_out : Outport  Port=2
  wires:
  I_in/1 -> C_cable/2(I_in)
  Gain/1 -> Add1/2
  Add1/1 -> C_cable/1(It2)
  Transformer-complex/2(It2) -> Add1/1
  V_in/1 -> Cable line/1(V)
  Transformer-complex/1(It1) -> C_filter/2(It1)
  Cable line/1(I_cable) -> C_cable/3(I_cable), I_out/1
  C_filter/1(Vc_filter_qd) -> Transformer-complex/1(Vc_filter_qd), WFG/1(vcqd)
  WFG/1(ilqd) -> C_filter/1(IWF)
  C_cable/1(Vc_cable) -> Cable line/2(Vc_cable), V_out/1, Transformer-complex/2(Vc_cable_qd), Gain/1

== system wind1000_BUS_Original/Subsystem_200/Subsystem/String20/WT8 ==
  V_in : Inport
  I_in : Inport  Port=2
  Add1 : Sum  Inputs=+-
  C_cable : Subsystem
  C_filter : Subsystem
  Cable line : Subsystem
  Gain : Gain  Gain=1/parGrid.WTstring.Rparallel_cable
  Transformer-complex : Subsystem
  WFG : Subsystem
  V_out : Outport
  I_out : Outport  Port=2
  wires:
  C_cable/1(Vc_cable) -> Gain/1, Transformer-complex/2(Vc_cable_qd), V_out/1, Cable line/2(Vc_cable)
  WFG/1(ilqd) -> C_filter/1(IWF)
  C_filter/1(Vc_filter_qd) -> Transformer-complex/1(Vc_filter_qd), WFG/1(vcqd)
  Cable line/1(I_cable) -> I_out/1, C_cable/3(I_cable)
  Transformer-complex/1(It1) -> C_filter/2(It1)
  V_in/1 -> Cable line/1(V)
  Transformer-complex/2(It2) -> Add1/1
  Add1/1 -> C_cable/1(It2)
  Gain/1 -> Add1/2
  I_in/1 -> C_cable/2(I_in)
